simulink model "HDVSim_XNG_Hybrid_Truck"  (R2019b)
settings: Solver=ode3  StartTime=0.0  StopTime=inf  FixedStep=sample_time  MaxStep=0.1  RelTol=1e-3  EnableMultiTasking=on
model workspace (MAT data in the model file):
  Anschaffungskosten = [3707764736; 2; 1; 1; 1; 1]
  Bat.Useable_capacity = 27
  Bat.Useable_range = 0.32
  Bat.Type = 2
  Bat.SOC_start = 1
  Bat.Voltage = 800
  Bat.Charge_cycles = 13055.4976829
  Bat.Efficiency_power_electronics = 0.95
  DrvTrn = 4
  Eigenschaften = [3707764736; 2; 1; 1; 2; 2]
  Electric_Truck = 0
  Elektro_LKW = 0
  Fahrzyklus = 5
  Fueltype = 6
  Gewichte = [3707764736; 2; 1; 1; 3; 3]
  Hybrid_LKW = 1
  Hybrid_Truck = 1
  IZ12 = 3600
  Kosten = [3707764736; 2; 1; 1; 3; 3]
  Kraftstoff = 6
  SOC_T_EM_completely_available = 0.448
  SOC_addition = 0.2
  SOC_soll = 0.5
  SOC_target = 0.05
  Sailing_without_drag_torque = 0
  TCO = TCO
  TCO.Ratio_Trailer_Truck = [1 1]
  TCO.Purchase_price = [113872 59191]
  TCO.Price_increase_repurchase = [3.5 3.5]
  TCO.Replacement_original_price = [77010 54183]
  TCO.Residual_value = [34162 0]
  TCO.bDiesel = 0
  TCO.CNG_consumption = 0
  TCO.LNG_consumption = 23.6968877169
  TCO.energyTotal = 19.9299848679
  TCO.Hydrogen_consumption = 0
  TCO.Tire_costs_set = [2700 5008]
  TCO.Amortization_value = [94665 81874]
  TCO.Toll_rate = 0.135
  TCO.Daily_working_time = 8
  TCO.Other_works_day = 0.5
  TCO.Gross_hourly_wage_driver = 13
  TCO.Operating_days_year = 240
  TCO.Annual_gross_wage_driver = 24960
  TCO.Personnel_factor = 1.3
  TCO.Annual_gross_wage_truck = 32448
  TCO.Social_costs_percent = 25.75
  TCO.Social_costs_EUR = 8355
  TCO.Back_up_driver = 0
  TCO.Fees_day = 12
  TCO.Fees_year = 2880
  TCO.Driver_costs_other = 0
  TCO.Driving_personnel_costs_total = 43683
  TCO.Diesel_price = 0.95
  TCO.Diesel_price_external = 1
  TCO.Adblue_price = 0.68
  TCO.CNG_price = 0.88
  TCO.LNG_price = 0.81
  TCO.Electricity_price = 0.12
  TCO.Hydrogen_price = 9
  TCO.Interest_current_asset = 8.4
  TCO.Interest_fixed_asset = 8.4
  TCO.Administrative_costs = 10.7
  TCO.Driving_distance = 108.251724403
  TCO.Ratio_toll_route = 75
  TCO.Loading_time_combination = 0.25
  TCO.Unloading_time_combination = 0.25
  TCO.Ratio_empty_trips = 0
  TCO.Average_speed = 78.4432785528
  TCO.Daily_driving_time = 5.51
  TCO.Annual_mileage = [103733 103733]
  TCO.Operating_life = [6 12]
  TCO.Tire_mileage = [140000 170000]
  TCO.Ratio_inhouse_tanking_diesel = 75
  TCO.Lubricant_consumption = 1
  TCO.Adblue_consumption = 4
  TCO.Afa_time_dependent = [100 100]
  TCO.Afa_power_dependent = [0 0]
  TCO.Current_asset = [6000 1400]
  TCO.Taxes = [556 373]
  TCO.Insurance_vehicle = [6298 677]
  TCO.Insurance_transport = 1000
  TCO.Communication = 600
  TCO.Repair_maintenance_care = [0.13 0.025]
  TCO.Amortisation_time_dependent = [15778 6823]
  TCO.Current_assets_interest_bearing = 622
  TCO.Fixed_assets_interest_bearing = [4783 2486]
  TCO.Insurance = [7298 677]
  TCO.Sum_vehicle_costs = [29637 10359]
  TCO.Sum_fix_costs = [73320 10359]
  TCO.Amortisation_performance_related = [0 0]
  TCO.Fuel_costs = 22392
  TCO.Lubricant_costs = 199
  TCO.Adblue_costs = 0
  TCO.Repair_costs = [13485 2593]
  TCO.Tire_costs = [2001 3056]
  TCO.Lebensdauer_Batteriepack = 7.12416466393
  TCO.Anschaffungspreis_Akkupack = 19520.8885872
  TCO.Verschleisskosten_Batteriepack = 0
  TCO.Sum_variable_costs = [38077 5649]
  TCO.Sum_fix_variable_costs = [111397 16008]
  TCO.Overhead_costs = 15266
  TCO.Toll_costs = 10502.96625
  TCO.Total_costs = 153173.96625
  TCO.Results = table
  TCO.Results.data = {{[]; []; []; []; []; []; []; []; []; []; []; []; []; []; []; []; []; []; []; []; []; []; []}, {[]; 0.217876664128; 0.00599616322675; 0.070074132629; 0.0089556843049; 0.0768800670953; 0.00578408028303; 0.385566791667; 0.421109965006; 0.806676756673; []; 0; 0.215861876163; 0; 0.154994071318; 0.00191838662721; 0.0487501566522; 0.42152449076; []; 1.22820124743; 0.147166282668; 0.10125; 1.4766175301}, {[]; []; []; []; []; []; []; []; []; []; []; []; []; []; []; []; []; []; []; []; []; []; []}, {'#cccccc'; '#9DFF00'; '#CEFF00'; '#FFFF00'; '#FFDF00'; '#FFB900'; '#FF9200'; '#FF5D00'; '#FF7F7F'; '#FF2900'; '#cccccc'; '#00FF85'; '#00FFC6'; '#00FFF2'; '#00FFFF'; '#00DBFF'; '#00B3FF'; '#0088FF'; '#cccccc'; '#B100FF'; '#FF00FF'; '#FF00C0'; '#FF0070'}, [0; 1; 1; 1; 1; 1; 1; 0; 1; 0; 0; 1; 1; 1; 1; 1; 1; 0; 0; 0; 1; 1; 0], {[]; 22601; 622; 7269; 929; 7975; 600; 39996; 43683; 83679; []; 0; 22392; 0; 16078; 199; 5057; 43726; []; 127405; 15266; 10502.96625; 153173.96625}}
  TCO.Results.ndims = 2
  TCO.Results.nrows = 23
  TCO.Results.rownames = {'Time_dependent_vehicle_costs'; 'Amortisation_time_dependent'; 'Current_assets_interest_bearing'; 'Fixed_assets_interest_bearing'; 'Taxes'; 'Insurance'; 'Communication'; 'Sum_vehicle_costs'; 'Driving_personnel_costs_total'; 'Sum_fix_costs'; 'Performance_related_vehicle_costs'; 'Amortisation_performance_related'; 'Fuel_costs'; 'Adblue_costs'; 'Repair_costs'; 'Lubricant_costs'; 'Tire_costs'; 'Sum_variable_costs'; 'Sum_costs'; 'Sum_fix_variable_costs'; 'Overhead_costs'; 'Toll_costs'; 'Total_costs'}
  TCO.Results.nvars = 6
  TCO.Results.varnames = {'Euro_Year', 'Euro_km', 'Percent', 'Cellcolor', 'Diagramm', 'Euro_Jahr'}
  TCO.Results.props.CustomProps = struct()
  TCO.Results.props.VariableCustomProps = struct()
  TCO.Results.props.versionSavedFrom = 4
  TCO.Results.props.minCompatibleVersion = 1
  TCO.Results.props.incompatibilityMsg = ''
  TCO.Results.props.VersionSavedFrom = 4
  TCO.Results.props.Description = ''
  TCO.Results.props.useVariableNamesOriginal = 0
  TCO.Results.props.useDimensionNamesOriginal = 0
  TCO.Results.props.VariableNamesOriginal = {}
  TCO.Results.props.DimensionNames = {'Row', 'Variables'}
  TCO.Results.props.DimensionNamesOriginal = {}
  TCO.Results.props.UserData = []
  TCO.Results.props.VariableDescriptions = {}
  TCO.Results.props.VariableUnits = {}
  TCO.Results.props.VariableContinuity = {}
  TCO_Anhaenger = 1
  TCO_Trailer = 1
  T_distance_LPS_down = 141
  T_distance_LPS_up = 366
  VSim.Display = 3
  VSim.Opt = 0
  VSim.v_t = [0 0 0 0 0 0 7.78702558964 13.5455486475 17.5098763901 20.64771434 22.6611629891 24.5808920247 26.497930615 27.9436285122 29.2700076923 30.5941752972 31.916035258 33.1908328261 34.0616736486 34.9308240914 35.7982444287 36.663895271 37.5277375709 38.3897326301 39.2498421055 40.1080280155 40.9642527457 41.8184790552 42.6706700821 43.4527673116 43.9611797576 44.4683183246 44.9741715644 45.4787281689 45.9819769713 46.4839069461 47.9488358042 49.5523927745 51.1896098137 52.8609582212 54.5660311627 56.2956841938 58.0503332316 59.8108324205 61.5662383358 63.3222898223 65.0739909595 66.8139377448 68.5280042906 70.2103693282 71.8624252942 73.4760089439 75.0504359591 76.5862765506 78.0850203676 79.5458647769 80.9710388101 81.5966525882 80.8908314832 81.9503579711 83.0914322002 84.2274160693 85.3582506742 86.2443475854 86.4930083388 86.555730356 86.5740585846]
  Vehicle = 'LNGHybrid'
  WPT.Voltage = 0
  WPT.P_max = 200
  WPT.expansion = 0.3
  WPT.eta = 0.92
  WPT.SOC_target = 0.8
  WPT.SOC_electric_only = 0.3
  Zugmaschinengewicht = 0
  acquisitionCosts = acquisitionCosts
  acquisitionCosts.KA_ZM = 113872.311812
  acquisitionCosts.KG = 5933.68525734
  acquisitionCosts.Getr_m = 256.411373638
  acquisitionCosts.KMot = 9881.5
  acquisitionCosts.Motor_Mmax = 1500
  acquisitionCosts.Motor_m = 846.7
  acquisitionCosts.KH = 15531.6957356
  acquisitionCosts.Hybrid = 1
  acquisitionCosts.Bat = 12080
  acquisitionCosts.Bat_type = 2
  acquisitionCosts.Bat_m = 419.254658385
  acquisitionCosts.Bat_kWh = 67.5
  acquisitionCosts.Ratio_BEV2PHEV = 1
  acquisitionCosts.EM = 1927.38569435
  acquisitionCosts.EM_Type = 2
  acquisitionCosts.EM_P_max = 187.306675836
  acquisitionCosts.LE = 842.88004126
  acquisitionCosts.KWPT = 10000
  acquisitionCosts.FC = 0
  acquisitionCosts.P_FC = 0
  acquisitionCosts.KA = 1563
  acquisitionCosts.v_diesel = 0
  acquisitionCosts.v_cng = 0
  acquisitionCosts.v_lng = 400
  acquisitionCosts.m_h2 = 0
  acquisitionCosts.KT = 4692.4
  acquisitionCosts.KTD = 0
  acquisitionCosts.KTCNG = 0
  acquisitionCosts.KTLNG = 4692.4
  acquisitionCosts.KTFC = 0
  acquisition_costs = [3707764736; 2; 1; 1; 3; 3]
  addition_for_critical_slope = 0.037
  ambient.gravity = 9.80665
  ambient.air_density = 1.1883
  ambient.friction_coeff = 0.8
  auxiliary_consumers.onBattery = 1
  auxiliary_consumers.power = 4859
  critical_altitude_difference = 50
  cycle.distance = [0 2e-05 0.25 0.6036 1.1529 1.9121 2.8899 4.092 5.1928 6.1952 7.4225 8.8723 ... (10385 elements, 10385x1)]
  cycle.speed = [0 0 16.6666666667 16.6666666667 16.6666666667 16.6666666667 16.6666666667 16.6666666667 16.6666666667 16.6666666667 16.6666666667 16.6666666667 ... (10385 elements, 10385x1)]
  cycle.slope = [0.029502 0.029502 0.029502 0.029502 0.029502 0.029502 0.029502 0.029502 0.029502 0.029502 0.029502 0.029502 ... (10385 elements, 10385x1)]
  cycle.stop_time = [1 1 0 0 0 0 0 0 0 0 0 0 ... (10385 elements, 10385x1)]
  cycle.altitude = [0 0 0 0 0 0 0 0 0 0 0 0 ... (10385 elements, 10385x1)]
  cycle.stop_end = 5
  cycle.stop_start = 5
  cycle.speed_init = 0
  cycle.delta12 = [0 0 0 0 0 0 0 0 0 0 0 0 ... (10385 elements, 1x10385)]
  cycle.delta23 = [0 0 0 0 0 0 0 0 0 0 0 0 ... (10385 elements, 1x10385)]
  dcycle = 20
  distance_1_PPC = 330
  distance_2_PPC = 340
  distance_PPC_altitude_difference = 4601
  driver.controller_proportional_gain = 230000
  driver.controller_integral_gain = 3000
  driver.slew_rate_throttle_in = 2
  driver.slew_rate_throttle_out = -2
  driver.slew_rate_brake_in = 2
  driver.slew_rate_brake_out = -2
  em.P_max = 187.306675836
  em.M_max = 1211
  em.n_eck = 1477
  em.n_max = 2000
  em.shift_parameter.n_pref = 1388.38
  em.shift_parameter.n2 = 310.17
  em.shift_parameter.n6 = 1600
  em.shift_parameter.M_max = 1211
  em.fuel.co2_per_kwh = 504
  em.speed = [0 10 20 30 40 50 60 70 80 90 100 110 120 130 140 150 160 170 180 190 200 210 220 230 240 250 260 270 280 290 300 310 320 330 340 350 360 370 380 390 400 410 420 430 440 450 460 470 480 490 500 510 520 530 540 550 560 570 580 590 600 610 620 630 640 650 660 670 680 690 700 710 720 730 740 750 760 770 780 790 800 810 820 830 840 850 860 870 880 890 900 910 920 930 940 950 960 970 980 990 1000 1010 1020 1030 1040 1050 1060 1070 1080 1090 1100 1110 1120 1130 1140 1150 1160 1170 1180 1190 1200 1210 1220 1230 1240 1250 1260 1270 1280 1290 1300 1310 1320 1330 1340 1350 1360 1370 1380 1390 1400 1410 1420 1430 1440 1450 1460 1470 1480 1490 1500 1510 1520 1530 1540 1550 1560 1570 1580 1590 1600 1610 1620 1630 1640 1650 1660 1670 1680 1690 1700 1710 1720 1730 1740 1750 1760 1770 1780 1790 1800 1810 1820 1830 1840 1850 1860 1870 1880 1890 1900 1910 1920 1930 1940 1950 1960 1970 1980 1990 2000]
  em.trq = [1211 1211 1211 1211 1211 1211 1211 1211 1211 1211 1211 1211 1211 1211 1211 1211 1211 1211 1211 1211 1211 1211 1211 1211 1211 1211 1211 1211 1211 1211 1211 1211 1211 1211 1211 1211 1211 1211 1211 1211 1211 1211 1211 1211 1211 1211 1211 1211 1211 1211 1211 1211 1211 1211 1211 1211 1211 1211 1211 1211 1211 1211 1211 1211 1211 1211 1211 1211 1211 1211 1211 1211 1211 1211 1211 1211 1211 1211 1211 1211 1211 1211 1211 1211 1211 1211 1211 1211 1211 1211 1211 1211 1211 1211 1211 1211 1211 1211 1211 1211 1211 1211 1211 1211 1211 1211 1211 1211 1211 1211 1211 1211 1211 1211 1211 1211 1211 1211 1211 1211 1211 1211 1211 1211 1211 1211 1211 1211 1211 1211 1211 1211 1211 1211 1211 1211 1211 1211 1211 1211 1211 1211 1211 1211 1211 1211 1211 1211 1208.54527027 1200.43422819 1192.43133333 1184.53443709 1176.74144737 1169.0503268 1161.45909091 1153.96580645 1146.56858974 1139.2656051 1132.05506329 1124.93522013 1117.904375 1110.96086957 1104.10308642 1097.32944785 1090.63841463 1084.02848485 1077.49819277 1071.04610778 1064.67083333 1058.37100592 1052.14529412 1045.99239766 1039.91104651 1033.9 1027.95804598 1022.084 1016.27670455 1010.53502825 1004.85786517 999.244134078 993.692777778 988.202762431 982.773076923 977.40273224 972.09076087 966.836216216 961.638172043 956.495721925 951.407978723 946.374074074 941.393157895 936.464397906 931.586979167 926.760103627 921.982989691 917.254871795 912.575 907.942639594 903.357070707 898.81758794 894.3235]
  em.Type = 2
  em.efficiency.characteristic_map = [0.001 0.001 0.001 0.001 0.001 0.001 0.001 0.001 0.001 0.001 0.001 0.001 ... (20301 elements, 101x201)]
  em.efficiency.speed = [0 10 20 30 40 50 60 70 80 90 100 110 120 130 140 150 160 170 180 190 200 210 220 230 240 250 260 270 280 290 300 310 320 330 340 350 360 370 380 390 400 410 420 430 440 450 460 470 480 490 500 510 520 530 540 550 560 570 580 590 600 610 620 630 640 650 660 670 680 690 700 710 720 730 740 750 760 770 780 790 800 810 820 830 840 850 860 870 880 890 900 910 920 930 940 950 960 970 980 990 1000 1010 1020 1030 1040 1050 1060 1070 1080 1090 1100 1110 1120 1130 1140 1150 1160 1170 1180 1190 1200 1210 1220 1230 1240 1250 1260 1270 1280 1290 1300 1310 1320 1330 1340 1350 1360 1370 1380 1390 1400 1410 1420 1430 1440 1450 1460 1470 1480 1490 1500 1510 1520 1530 1540 1550 1560 1570 1580 1590 1600 1610 1620 1630 1640 1650 1660 1670 1680 1690 1700 1710 1720 1730 1740 1750 1760 1770 1780 1790 1800 1810 1820 1830 1840 1850 1860 1870 1880 1890 1900 1910 1920 1930 1940 1950 1960 1970 1980 1990 2000]
  em.efficiency.torque = [0 12.11 24.22 36.33 48.44 60.55 72.66 84.77 96.88 108.99 121.1 133.21 145.32 157.43 169.54 181.65 193.76 205.87 217.98 230.09 242.2 254.31 266.42 278.53 290.64 302.75 314.86 326.97 339.08 351.19 363.3 375.41 387.52 399.63 411.74 423.85 435.96 448.07 460.18 472.29 484.4 496.51 508.62 520.73 532.84 544.95 557.06 569.17 581.28 593.39 605.5 617.61 629.72 641.83 653.94 666.05 678.16 690.27 702.38 714.49 726.6 738.71 750.82 762.93 775.04 787.15 799.26 811.37 823.48 835.59 847.7 859.81 871.92 884.03 896.14 908.25 920.36 932.47 944.58 956.69 968.8 980.91 993.02 1005.13 1017.24 1029.35 1041.46 1053.57 1065.68 1077.79 1089.9 1102.01 1114.12 1126.23 1138.34 1150.45 1162.56 1174.67 1186.78 1198.89 1211]
  em.noEM = 2
  em_M_max = 797.415556
  engine.bsfc.be = [0.131482188876 0.133620299042 0.135839892639 0.138147190922 0.140549191494 0.143053789211 0.145669914251 0.148407687189 0.151278588529 0.154295635515 0.157473550327 0.160828886947 ... (38000 elements, 190x200)]
  engine.bsfc.trqorig = [0 5.95509049885 11.9101809977 17.8652714966 23.8203619954 29.7754524943 35.7305429931 41.685633492 47.6407239908 53.5958144897 59.5509049885 65.5059954874 ... (190 elements, 190x1)]
  engine.bsfc.speed = [798.227848101 805.263023981 812.29819986 819.333375739 826.368551619 833.403727498 840.438903378 847.474079257 854.509255136 861.544431016 868.579606895 875.614782775 ... (200 elements, 1x200)]
  engine.bsfc.be_min = 0.372504791257
  engine.bsfc.M_be_min_orig = [684.835407368 690.790497867 696.745588366 702.700678865 708.655769363 714.610859862 720.565950361 732.476131359 738.431221858 744.386312357 750.341402855 756.296493354 ... (200 elements, 1x200)]
  engine.bsfc.trq = [0 7.97556763239 15.9511352648 23.9267028972 31.9022705296 39.877838162 47.8534057943 55.8289734267 63.8045410591 71.7801086915 79.7556763239 87.7312439563 ... (190 elements, 190x1)]
  engine.bsfc.M_be_min = [917.190277725 925.165845357 933.14141299 941.116980622 949.092548255 957.068115887 965.043683519 980.994818784 988.970386417 996.945954049 1004.92152168 1012.89708931 ... (200 elements, 1x200)]
  engine.full_load.trqorig = [684.835407368 690.790497867 696.745588366 702.700678865 708.655769363 714.610859862 720.565950361 732.476131359 738.431221858 744.386312357 750.341402855 756.296493354 ... (200 elements, 1x200)]
  engine.full_load.speed = [798.227848101 805.263023981 812.29819986 819.333375739 826.368551619 833.403727498 840.438903378 847.474079257 854.509255136 861.544431016 868.579606895 875.614782775 ... (200 elements, 1x200)]
  engine.full_load.trq = [917.190277725 925.165845357 933.14141299 941.116980622 949.092548255 957.068115887 965.043683519 980.994818784 988.970386417 996.945954049 1004.92152168 1012.89708931 ... (200 elements, 1x200)]
  engine.full_load.power = [76.6681414837 78.0164109099 79.3764318868 80.7482044142 82.1317284922 83.5270041209 84.9340313002 87.0606199483 88.4970260041 89.9451836104 91.4050927674 92.876753475 ... (200 elements, 1x200)]
  engine.number = 3
  engine.M_max_original = 1120
  engine.M_max = 1500
  engine.scale_factor = 1.33928571429
  engine.speed_min = 800
  engine.speed_max = 1900
  engine.idle_fuel_consumption = 1.5
  engine.drag_torque.trq = [-160.714285714 -348.214285714]
  engine.drag_torque.speed = [600 2190]
  engine.shift_parameter.n_lo = 1000
  engine.shift_parameter.n_pref = 1279
  engine.shift_parameter.n1 = 600
  engine.shift_parameter.n2 = 800
  engine.shift_parameter.n3 = 1139.5
  engine.shift_parameter.n4 = 800
  engine.shift_parameter.n5 = 1279
  engine.shift_parameter.n6 = 1900
  engine.shift_parameter.M_max = 1499.40671489
  engine.fuel.density = 424
  engine.fuel.co2_per_kg = 2.75
  engine.fuel.heat_of_combustion = 50000
  engine.fuel.Gasart = 'LNG'
  final_drive.ratio = 2.884
  final_drive.trq_eff = 0.98
  ifElectric = 0
  ifOptimized = 1
  look_ahead_PPC = 710
  max_distance = 108191.00003
  name = 'HDVSim_XNG_Hybrid_Truck'
  preditiveCruiseControl = 0
  propertie = [3707764736; 2; 1; 1; 4; 4]
  prospective_cruise_control = 1
  sample_time = 0.1
  tank.v_diesel = 0
  tank.v_cng = 0
  tank.v_lng = 400
  tank.m_h2 = 0
  tires.radius = 0.4465
  tires.roll_drag_coeff.total = 0.007
  transmission.init_gear = 1
  transmission.shift_time = 1
  transmission.ratios = [11.689 8.8947844856 6.76851664345 5.15052586455 3.91931025346 2.98241252774 2.26947700243 1.72696627869 1.31414088997 1]
  transmission.trq_eff = [0.96 0.96 0.96 0.96 0.98 0.97 0.97 0.97 0.97 0.99]
  transmission.lamda = [1.10949792808 1.06119976752 1.03288013965 1.02166016924 1.01558545301 1.01166143122 1.00954352608 1.0082007006 1.00714498093 1.006050163]
  transmission.z = 10
  v_PPC_delta = 2.77777777778
  v_max_electrical_drive_only = 12.8888888889
  vehicle.long_truck = 0
  vehicle.payload = 19300
  vehicle.frontal_area = 10.2
  vehicle.air_drag_coeff = 0.55
  vehicle.Fx_max = 90221.18
  vehicle.F_brake_max = 309890.14
  vehicle.mass = 13055.4017043
  vehicleProperties = vehicleProperties
  vehicleProperties.CO2_EM_Range = 100
  vehicleProperties.a_0_80_Range = 54
  vehicleProperties.BOK_ak = 2.55
  vehicleProperties.BOK_Range = 4.65
  vehicleProperties.V_Rel_ak = 0.55
  vehicleProperties.V_Rel_Range = 0.35
  vehicleProperties.VWF_ak = 0.9
  vehicleProperties.VWF_Range = 7.2
  vehicle_F_brake_max = 309890.14
  weights = weights
  weights.m_Engine = 846.7
  weights.m_Gearbox = 256.411373638
  weights.m_Retarder = 82
  weights.m_tank_system = 252.803932883
  weights.m_Fuel = 216
  weights.m_Exhaust = 118.57
  weights.m_Battery = 419.254658385
  weights.m_EM = 208.118528706
  weights.m_PwrElectr = 17.3432107255
  weights.m_Charger = 15
  weights.m_Trailer = 5400
  weights.m_Total = 7655.40170434
  weights.m_Base = 5223.2
  weights.Hybrid = 1
  weights.max_Payload = 27944.5982957
  weights.m_Max = 41000
  x = [11.689 7 0 0 2.884 1500 1000 1279 10 62 24 25 1211 1477 27 0.05 0.448 46.4 366 141 0.037 4601 50 0.2 0.32 2 2]
values from neighbouring files (symbols this model uses):
  transmission = Transmission_gearing(15.86, 7, 0, 0, final_drive.ratio)    [Parameterizing.m]
undefined symbols (not defined in the file or in its folder): Ion, Lithium, Vorausschauender_Tempomat
libraries: simulink x22, powerlib x2, electricdrivelib x1
(part 1 of 3)
settings Model: Creator=Alexander Suessmann  LastModifiedBy=ga73rep
settings DataIOCC: SaveFormat=Array  SignalLoggingSaveFormat=ModelDataLogs  SaveOutput=off  SignalLogging=off  DSMLogging=off  ReturnWorkspaceOutputs=off  SignalLoggingName=datalog
settings OptimizationCC: ExpressionDepthLimit=2147483647  InitFltsAndDblsToZero=on  LifeSpan=inf
settings DebuggingCC: UnderspecifiedInitializationDetection=Classic  MergeDetectMultiDrivingBlocksExec=none  SolverPrmCheckMsg=warning  MultiTaskDSMMsg=warning  MultiTaskCondExecSysMsg=none  FcnCallInpInsideContextMsg=warning  AllowSymbolicDim=off  StateNameClashWarn=warning  SFUnexpectedBacktrackingDiag=warning  SFNoUnconditionalDefaultTransitionDiag=warning  SFExecutionAtInitializationDiag=none  ArithmeticOperatorsInVariantConditions=warning
settings HardwareCC: ProdBitPerPointer=32  ProdBitPerSizeT=32  ProdBitPerPtrDiffT=32  ProdLargestAtomicFloat=None  ProdIntDivRoundTo=Undefined  ProdEndianess=Unspecified  ProdWordSize=32  ProdHWDeviceType=32-bit Generic
settings ModelReferenceCC: PropagateSignalLabelsOutOfModel=off
settings SFSimCC: SimUseLocalCustomCode=off  CustomCodeUndefinedFunction=UseInterfaceOnly
settings RTWCC: RTWUseLocalCustomCode=off
settings CodeAppCC: StateflowObjectComments=on
settings GRTTargetCC: TargetLangStandard=C89/C90 (ANSI)  GeneratePreprocessorConditionals=Disable all  CombineOutputUpdateFcns=off  GRTInterface=on
settings ConfigComp: CovMetricSettings=dw  CovHtmlReporting=on  CovSaveCumulativeToWorkspaceVar=on  CovSaveSingleToWorkspaceVar=on  CovExternalEMLEnable=off
settings SimscapeCC: SimscapeNormalizeSystem=off

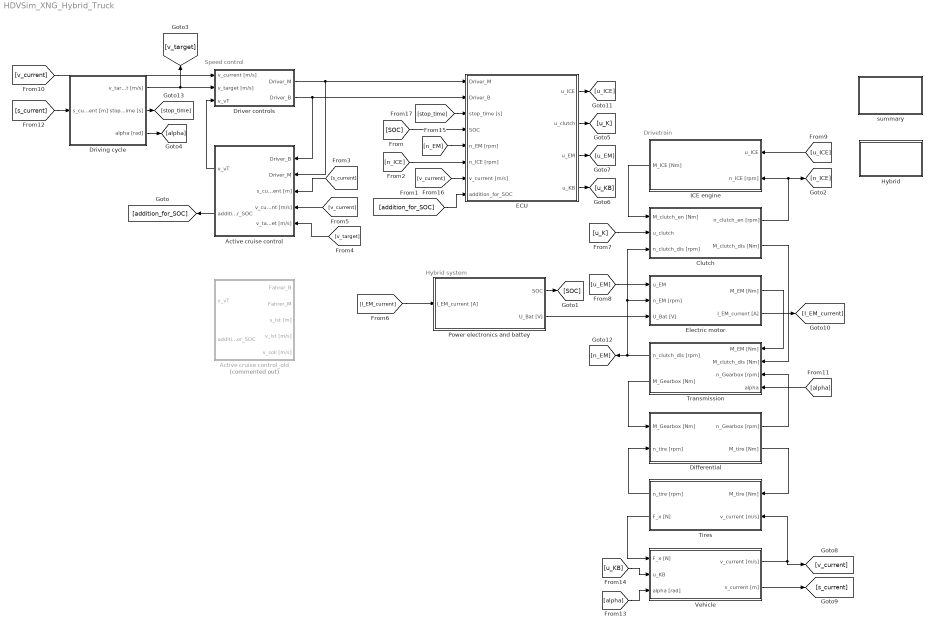
== system HDVSim_XNG_Hybrid_Truck ==
  note: Speed control
  note: Drivetrain
  note: Hybrid system
  Active cruise control : Subsystem
  Active cruise control_old : Subsystem  Commented=on
  Clutch : Subsystem
  Differential : Subsystem
  Driver controls : Subsystem
  Driving cycle : Subsystem
  ECU : Subsystem
  Electric motor : Subsystem
  Hybrid : Subsystem
  ICE engine : Subsystem
  Power electronics and battey : Subsystem
  Tires : Subsystem
  Transmission : Subsystem
  Vehicle : Subsystem
  summary : Subsystem
  wires:
  ICE engine/1(M_ICE [Nm]) -> Clutch/1(M_clutch_en [Nm])
  Transmission/1(n_clutch_dis [rpm]) -> [n_EM], Clutch/3(n_clutch_dis [rpm]), Electric motor/2(n_EM [rpm])
  Transmission/2(M_Gearbox [Nm]) -> Differential/1(M_Gearbox [Nm])
  Tires/1(n_tire [rpm]) -> Differential/2(n_tire [rpm])
  Clutch/1(n_clutch_en [rpm]) -> [n_ICE], ICE engine/2(n_ICE [rpm])
  Clutch/2(M_clutch_dis [Nm]) -> Transmission/2(M_clutch_dis [Nm])
  Differential/1(n_Gearbox [rpm]) -> Transmission/3(n_Gearbox [rpm])
  Differential/2(M_tire [Nm]) -> Tires/1(M_tire [Nm])
  Vehicle/1(v_current [m/s]) -> [v_current], Tires/2(v_current [m/s])
  Tires/2(F_x [N]) -> Vehicle/1(F_x [N])
  Electric motor/1(M_EM [Nm]) -> Transmission/1(M_EM [Nm])
  Driving cycle/1(v_target [m/s]) -> [v_target], Driver controls/2(v_target [m/s])
  Driving cycle/3(alpha [rad]) -> [alpha]
  Active cruise control/2(addition_for_SOC) -> [addition_for_SOC]
  Active cruise control/1(v_vT) -> Driver controls/3(v_vT)
  [s_current] -> Driving cycle/1(s_current [m])
  [v_current] -> Driver controls/1(v_current [m/s])
  [n_ICE] -> ECU/6(n_ICE [rpm])
  [SOC] -> ECU/4(SOC)
  [n_EM] -> ECU/5(n_EM [rpm])
  [v_current] -> ECU/7(v_current [m/s])
  [addition_for_SOC] -> ECU/8(addition_for_SOC)
  Driver controls/1(Driver_M) -> Active cruise control/2(Driver_M), ECU/1(Driver_M)
  Driver controls/2(Driver_B) -> Active cruise control/1(Driver_B), ECU/2(Driver_B)
  [s_current] -> Active cruise control/3(s_current [m])
  [v_current] -> Active cruise control/4(v_current [m/s])
  [v_target] -> Active cruise control/5(v_target [m/s])
  [I_EM_current] -> Power electronics and battey/1(I_EM_current [A])
  ECU/1(u_ICE) -> [u_ICE]
  ECU/2(u_clutch) -> [u_K]
  ECU/3(u_EM) -> [u_EM]
  ECU/4(u_KB) -> [u_KB]
  [u_K] -> Clutch/2(u_clutch)
  [u_EM] -> Electric motor/1(u_EM)
  Power electronics and battey/1(SOC) -> [SOC]
  Power electronics and battey/2(U_Bat [V]) -> Electric motor/3(U_Bat [V])
  [u_ICE] -> ICE engine/1(u_ICE)
  Electric motor/2(I_EM_current [A]) -> [I_EM_current]
  [alpha] -> Transmission/4(alpha)
  Vehicle/2(s_current [m]) -> [s_current]
  [u_KB] -> Vehicle/2(u_KB)
  [alpha] -> Vehicle/3(alpha [rad])
  Driving cycle/2(stop_time [s]) -> [stop_time]
  [stop_time] -> ECU/3(stop_time [s])

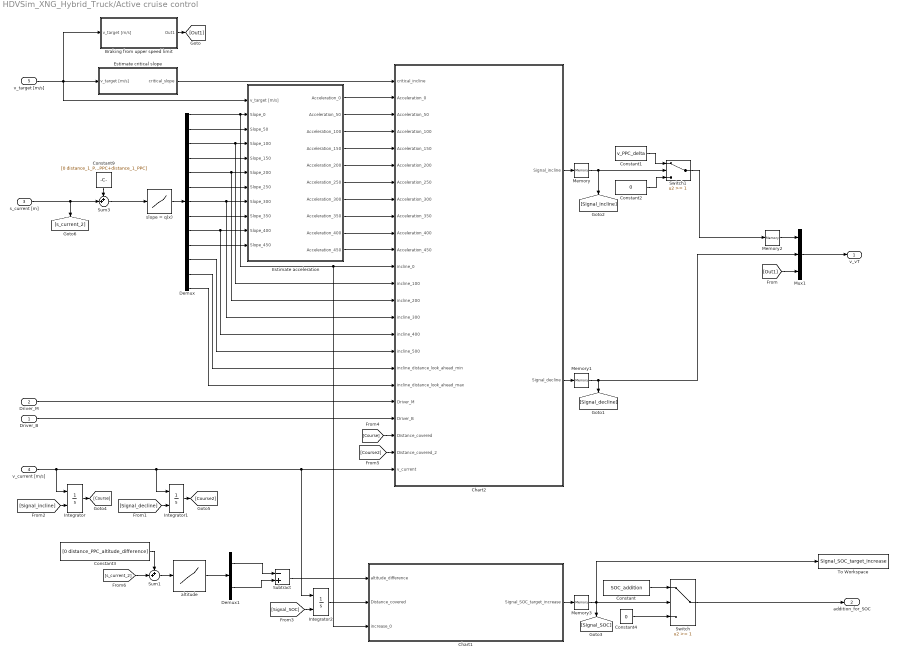
== system HDVSim_XNG_Hybrid_Truck/Active cruise control ==
  Driver_B : Inport
  Driver_M : Inport  Port=2
  s_current [m] : Inport  Port=3
  v_current [m/s] : Inport  Port=4
  v_target [m/s] : Inport  Port=5
  Braking from upper speed limit : Subsystem
  Chart1 : Stateflow chart  TreatAsAtomicUnit=on
  Chart2 : Stateflow chart  TreatAsAtomicUnit=on
  Constant : Constant  Value=SOC_addition
  Constant1 : Constant  Value=v_PPC_delta
  Constant2 : Constant  Value=0
  Constant3 : Constant  Value=[0 distance_PPC_altitude_difference]
  Constant4 : Constant  Value=0
  Constant9 : Constant  Value=[0 distance_1_PPC/5 2*distance_1_PPC/5 3*distance_1_PPC/5 4*distance_1_PPC/5 distance_1_PPC 6*distance_1_PPC/5 7*distance_1_PPC/5 8*distance_1_PPC/5 9*distance_1_PPC/5 10*distance_1_PPC/5 look_ahead_PPC look_ahead_PPC+distance_1_PPC]
  Demux : Demux  Outputs=13
  Demux1 : Demux  Outputs=2
  Estimate acceleration : Subsystem
  Estimate critical slope : Subsystem
  Integrator : Integrator  ExternalReset=rising
  Integrator1 : Integrator  ExternalReset=rising
  Integrator2 : Integrator  ExternalReset=rising
  Memory{,1..3} (4 alike) : Memory
  Mux1 : Mux  Inputs=3
  Subtract : Sum  Inputs=-+
  Sum1 : Sum  Inputs=++|
  Sum3 : Sum  Inputs=++|
  Switch : Switch  Threshold=1
  Switch1 : Switch  Threshold=1
  To Workspace : ToWorkspace  VariableName=Signal_SOC_target_increase  SaveFormat=Structure With Time  SampleTime=-1
  altitude : Lookup_n-D  NumberOfTableDimensions=1  BreakpointsForDimension1=cycle.distance  Table=cycle.altitude  InterpMethod=Flat  RndMeth=Simplest  InputSameDT=on
  slope = q(x) : Lookup  InputValues=cycle.distance  Table=cycle.slope  LookUpMeth=Interpolation-Use End Values
  v_vT : Outport  VectorParamsAs1DForOutWhenUnconnected=off
  addition_for_SOC : Outport  Port=2  VectorParamsAs1DForOutWhenUnconnected=off
  wires:
  slope = q(x)/1 -> Demux/1
  Demux/1 -> Chart1/3(increase_0), Chart2/12(incline_0), Estimate acceleration/2(Slope_0)
  Estimate critical slope/1(critical_slope) -> Chart2/1(critical_incline)
  Chart2/1(Signal_incline) -> Memory/1
  v_current [m/s]/1 -> Integrator/1, Integrator1/1, Chart2/24(v_current), Integrator2/1
  Memory/1 -> [Signal_incline], Switch1/2
  Mux1/1 -> v_vT/1
  Constant9/1 -> Sum3/1
  Sum3/1 -> slope = q(x)/1
  Estimate acceleration/1(Acceleration_0) -> Chart2/2(Acceleration_0)
  Estimate acceleration/2(Acceleration_50) -> Chart2/3(Acceleration_50)
  Estimate acceleration/3(Acceleration_100) -> Chart2/4(Acceleration_100)
  Estimate acceleration/4(Acceleration_150) -> Chart2/5(Acceleration_150)
  Estimate acceleration/5(Acceleration_200) -> Chart2/6(Acceleration_200)
  Estimate acceleration/6(Acceleration_250) -> Chart2/7(Acceleration_250)
  Estimate acceleration/7(Acceleration_300) -> Chart2/8(Acceleration_300)
  Estimate acceleration/8(Acceleration_350) -> Chart2/9(Acceleration_350)
  Chart2/2(Signal_decline) -> Memory1/1
  Memory1/1 -> Mux1/2, [Signal_decline]
  Driver_B/1 -> Chart2/21(Driver_B)
  Estimate acceleration/9(Acceleration_400) -> Chart2/10(Acceleration_400)
  Estimate acceleration/10(Acceleration_450) -> Chart2/11(Acceleration_450)
  Driver_M/1 -> Chart2/20(Driver_M)
  Demux/2 -> Estimate acceleration/3(Slope_50)
  Demux/3 -> Chart2/13(incline_100), Estimate acceleration/4(Slope_100)
  Demux/4 -> Estimate acceleration/5(Slope_150)
  Demux/5 -> Chart2/14(incline_200), Estimate acceleration/6(Slope_200)
  Demux/6 -> Estimate acceleration/7("Slope_250 ")
  Demux/7 -> Chart2/15(incline_300), Estimate acceleration/8(Slope_300)
  Demux/8 -> Estimate acceleration/9(Slope_350)
  Demux/9 -> Chart2/16(incline_400), Estimate acceleration/10(Slope_400)
  Demux/10 -> Estimate acceleration/11(Slope_450)
  Demux/12 -> Chart2/18(incline_distance_look_ahead_min)
  Demux/13 -> Chart2/19(incline_distance_look_ahead_max)
  v_target [m/s]/1 -> Braking from upper speed limit/1(v_target [m/s]), Estimate acceleration/1(v_target [m/s]), Estimate critical slope/1(v_target [m/s])
  Memory2/1 -> Mux1/1
  Constant1/1 -> Switch1/1
  Constant2/1 -> Switch1/3
  Demux/11 -> Chart2/17(incline_500)
  Switch1/1 -> Memory2/1
  Constant3/1 -> Sum1/1
  s_current [m]/1 -> [s_current_2], Sum3/2
  Sum1/1 -> altitude/1
  altitude/1 -> Demux1/1
  Demux1/2 -> Subtract/2
  Demux1/1 -> Subtract/1
  Subtract/1 -> Chart1/1(altitude_difference)
  Chart1/1(Signal_SOC_target_increase) -> Memory3/1
  Integrator2/1 -> Chart1/2(Distance_covered)
  Constant/1 -> Switch/1
  Constant4/1 -> Switch/3
  Switch/1 -> addition_for_SOC/1
  Memory3/1 -> Switch/2, To Workspace/1, [Signal_SOC]
  [Signal_decline] -> Integrator1/2
  Integrator1/1 -> [Course2]
  Integrator/1 -> [Course]
  [Signal_incline] -> Integrator/2
  [Course] -> Chart2/22(Distance_covered)
  [Course2] -> Chart2/23(Distance_covered_2)
  [Signal_SOC] -> Integrator2/2
  [s_current_2] -> Sum1/2
  Braking from upper speed limit/1(Out1) -> [Out1]
  [Out1] -> Mux1/3

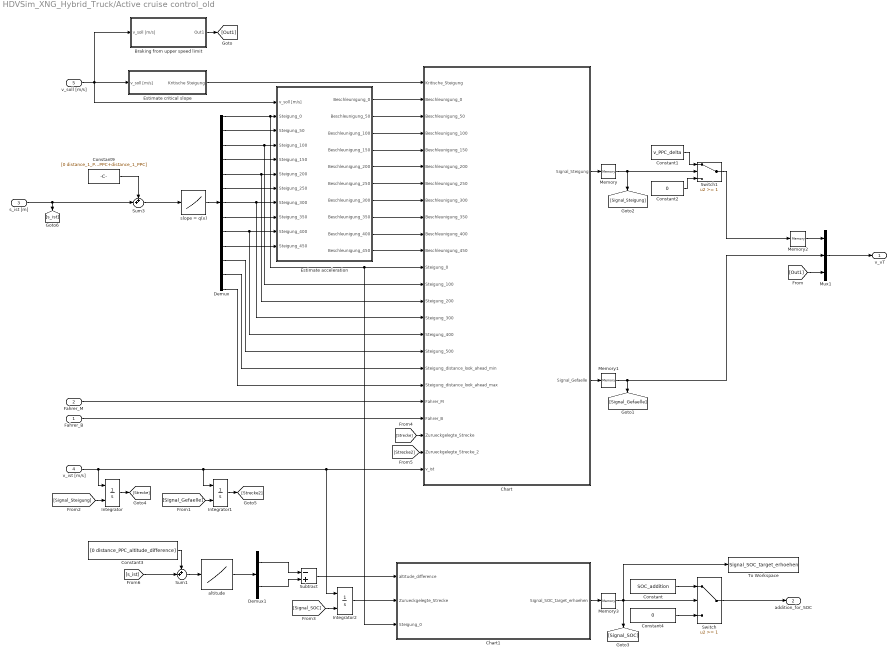
== system HDVSim_XNG_Hybrid_Truck/Active cruise control_old ==
  Fahrer_B : Inport
  Fahrer_M : Inport  Port=2
  s_ist [m] : Inport  Port=3
  v_ist [m/s] : Inport  Port=4
  v_soll [m/s] : Inport  Port=5
  Braking from upper speed limit : Subsystem
  Chart : Stateflow chart  TreatAsAtomicUnit=on
  Chart1 : Stateflow chart  TreatAsAtomicUnit=on
  Constant : Constant  Value=SOC_addition
  Constant1 : Constant  Value=v_PPC_delta
  Constant2 : Constant  Value=0
  Constant3 : Constant  Value=[0 distance_PPC_altitude_difference]
  Constant4 : Constant  Value=0
  Constant9 : Constant  Value=[0 distance_1_PPC/5 2*distance_1_PPC/5 3*distance_1_PPC/5 4*distance_1_PPC/5 distance_1_PPC 6*distance_1_PPC/5 7*distance_1_PPC/5 8*distance_1_PPC/5 9*distance_1_PPC/5 10*distance_1_PPC/5 look_ahead_PPC look_ahead_PPC+distance_1_PPC]
  Demux : Demux  Outputs=13
  Demux1 : Demux  Outputs=2
  Estimate acceleration : Subsystem
  Estimate critical slope : Subsystem
  Integrator : Integrator  ExternalReset=rising
  Integrator1 : Integrator  ExternalReset=rising
  Integrator2 : Integrator  ExternalReset=rising
  Memory{,1..3} (4 alike) : Memory
  Mux1 : Mux  Inputs=3
  Subtract : Sum  Inputs=-+
  Sum1 : Sum  Inputs=++|
  Sum3 : Sum  Inputs=++|
  Switch : Switch  Threshold=1
  Switch1 : Switch  Threshold=1
  To Workspace : ToWorkspace  VariableName=Signal_SOC_target_erhoehen  SaveFormat=Structure With Time  SampleTime=-1
  altitude : Lookup_n-D  NumberOfTableDimensions=1  BreakpointsForDimension1=cycle.distance  Table=cycle.altitude  InterpMethod=Flat  RndMeth=Simplest  InputSameDT=on
  slope = q(x) : Lookup  InputValues=cycle.distance  Table=cycle.slope  LookUpMeth=Interpolation-Use End Values
  v_vT : Outport  VectorParamsAs1DForOutWhenUnconnected=off
  addition_for_SOC : Outport  Port=2  VectorParamsAs1DForOutWhenUnconnected=off
  wires:
  slope = q(x)/1 -> Demux/1
  Demux/1 -> Chart1/3(Steigung_0), Chart/12(Steigung_0), Estimate acceleration/2(Steigung_0)
  Estimate critical slope/1(Kritische Steigung) -> Chart/1(Kritische_Steigung)
  Chart/1(Signal_Steigung) -> Memory/1
  v_ist [m/s]/1 -> Integrator2/1, Chart/24(v_ist), Integrator1/1, Integrator/1
  Memory/1 -> Switch1/2, [Signal_Steigung]
  Mux1/1 -> v_vT/1
  Constant9/1 -> Sum3/1
  Sum3/1 -> slope = q(x)/1
  Estimate acceleration/1(Beschleunigung_0) -> Chart/2(Beschleunigung_0)
  Estimate acceleration/2(Beschleunigung_50) -> Chart/3(Beschleunigung_50)
  Estimate acceleration/3(Beschleunigung_100) -> Chart/4(Beschleunigung_100)
  Estimate acceleration/4(Beschleunigung_150) -> Chart/5(Beschleunigung_150)
  Estimate acceleration/5(Beschleunigung_200) -> Chart/6(Beschleunigung_200)
  Estimate acceleration/6(Beschleunigung_250) -> Chart/7(Beschleunigung_250)
  Estimate acceleration/7(Beschleunigung_300) -> Chart/8(Beschleunigung_300)
  Estimate acceleration/8(Beschleunigung_350) -> Chart/9(Beschleunigung_350)
  Chart/2(Signal_Gefaelle) -> Memory1/1
  Memory1/1 -> Mux1/2, [Signal_Gefaelle]
  Fahrer_B/1 -> Chart/21(Fahrer_B)
  Estimate acceleration/9(Beschleunigung_400) -> Chart/10(Beschleunigung_400)
  Estimate acceleration/10(Beschleunigung_450) -> Chart/11(Beschleunigung_450)
  Fahrer_M/1 -> Chart/20(Fahrer_M)
  Demux/2 -> Estimate acceleration/3(Steigung_50)
  Demux/3 -> Chart/13(Steigung_100), Estimate acceleration/4(Steigung_100)
  Demux/4 -> Estimate acceleration/5(Steigung_150)
  Demux/5 -> Chart/14(Steigung_200), Estimate acceleration/6(Steigung_200)
  Demux/6 -> Estimate acceleration/7("Steigung_250 ")
  Demux/7 -> Chart/15(Steigung_300), Estimate acceleration/8(Steigung_300)
  Demux/8 -> Estimate acceleration/9(Steigung_350)
  Demux/9 -> Chart/16(Steigung_400), Estimate acceleration/10(Steigung_400)
  Demux/10 -> Estimate acceleration/11(Steigung_450)
  Demux/12 -> Chart/18(Steigung_distance_look_ahead_min)
  Demux/13 -> Chart/19(Steigung_distance_look_ahead_max)
  v_soll [m/s]/1 -> Braking from upper speed limit/1(v_soll [m/s]), Estimate critical slope/1(v_soll [m/s]), Estimate acceleration/1(v_soll [m/s])
  Memory2/1 -> Mux1/1
  Constant1/1 -> Switch1/1
  Constant2/1 -> Switch1/3
  Demux/11 -> Chart/17(Steigung_500)
  Switch1/1 -> Memory2/1
  Constant3/1 -> Sum1/1
  s_ist [m]/1 -> [s_ist], Sum3/2
  Sum1/1 -> altitude/1
  altitude/1 -> Demux1/1
  Demux1/2 -> Subtract/2
  Demux1/1 -> Subtract/1
  Subtract/1 -> Chart1/1(altitude_difference)
  Chart1/1(Signal_SOC_target_erhoehen) -> Memory3/1
  Integrator2/1 -> Chart1/2(Zurueckgelegte_Strecke)
  Constant/1 -> Switch/1
  Constant4/1 -> Switch/3
  Switch/1 -> addition_for_SOC/1
  Memory3/1 -> Switch/2, To Workspace/1, [Signal_SOC]
  Braking from upper speed limit/1(Out1) -> [Out1]
  [Out1] -> Mux1/3
  [Signal_Steigung] -> Integrator/2
  [Signal_SOC] -> Integrator2/2
  [Strecke] -> Chart/22(Zurueckgelegte_Strecke)
  Integrator/1 -> [Strecke]
  [Signal_Gefaelle] -> Integrator1/2
  Integrator1/1 -> [Strecke2]
  [Strecke2] -> Chart/23(Zurueckgelegte_Strecke_2)
  [s_ist] -> Sum1/2

== system HDVSim_XNG_Hybrid_Truck/Clutch ==
  note: Torque where clutch is disengaged
  note: Speed where clutch is engaged
  M_clutch_en [Nm] : Inport
  u_clutch : Inport  Port=2
  n_clutch_dis [rpm] : Inport  Port=3
  Compare To Zero : simulink/Logic and Bit Operations/Compare To Zero  relop===  OutDataTypeStr=boolean
  Constant : Constant  Value=0
  Idle engine speed : Constant  Value=engine.speed_min
  Logical Operator : Logic  AllPortsSameDT=off  OutDataTypeStr=boolean
  Lower engine speed : Saturate  UpperLimit=10000  LowerLimit=engine.speed_min  ZeroCross=off
  Switch1 : Switch  Threshold=1
  Switch2 : Switch  Threshold=1
  Switch3 : Switch  Threshold=1
  Zero1 : Constant  Value=0
  n_clutch_en [rpm] : Outport  VectorParamsAs1DForOutWhenUnconnected=off
  M_clutch_dis [Nm] : Outport  Port=2  VectorParamsAs1DForOutWhenUnconnected=off
  wires:
  n_clutch_dis [rpm]/1 -> Lower engine speed/1
  Lower engine speed/1 -> Switch3/3
  Switch2/1 -> Switch1/3
  Zero1/1 -> Switch2/1
  M_clutch_en [Nm]/1 -> Switch2/3
  [M_mot] -> Compare To Zero/1
  Compare To Zero/1 -> Logical Operator/1
  [ZKU] -> Logical Operator/2
  Logical Operator/1 -> Switch2/2
  Switch1/1 -> M_clutch_dis [Nm]/1
  Constant/1 -> Switch1/1
  Switch3/1 -> n_clutch_en [rpm]/1
  u_clutch/1 -> Switch3/2, Switch1/2
  Idle engine speed/1 -> Switch3/1

== system HDVSim_XNG_Hybrid_Truck/Differential ==
  note: Effective wheel torque
  M_Gearbox [Nm] : Inport
  n_tire [rpm] : Inport  Port=2
  Differential efficiency : Constant  Value=final_drive.trq_eff
  Differential gear ratio : Constant  Value=final_drive.ratio
  M' = M*i*eta : Product  Inputs=***
  Product : Product  Inputs=/  RndMeth=Zero
  Switch : Switch  ZeroCross=off
  n' *i = n : Product  RndMeth=Zero
  n_Gearbox [rpm] : Outport  VectorParamsAs1DForOutWhenUnconnected=off
  M_tire [Nm] : Outport  Port=2  VectorParamsAs1DForOutWhenUnconnected=off
  tag scope: Eta_Achsle_gearbox=global
  wires:
  M_Gearbox [Nm]/1 -> M' = M*i*eta/1, Switch/2
  n_tire [rpm]/1 -> n' *i = n/2
  Differential gear ratio/1 -> n' *i = n/1, M' = M*i*eta/3
  n' *i = n/1 -> n_Gearbox [rpm]/1
  M' = M*i*eta/1 -> M_tire [Nm]/1
  Differential efficiency/1 -> Product/1, Switch/1
  Product/1 -> Switch/3
  Switch/1 -> [Eta_Achsle_gearbox], M' = M*i*eta/2

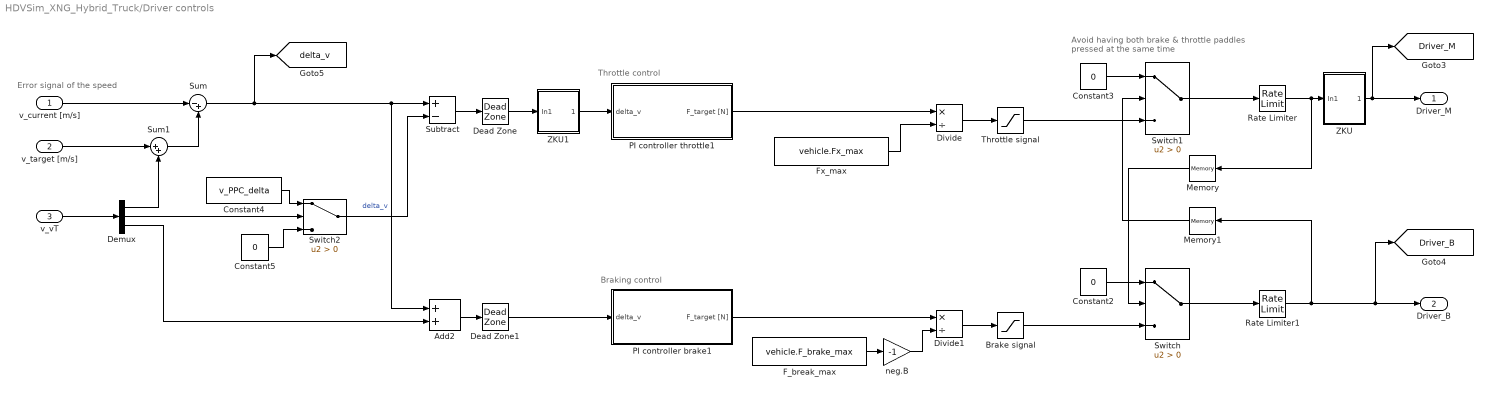
== system HDVSim_XNG_Hybrid_Truck/Driver controls ==
  note: Avoid having both brake & throttle
      | paddles pressed at the same time
  note: Throttle control
  note: Braking control
  note: Error signal of the speed
  v_current [m/s] : Inport
  v_target [m/s] : Inport  Port=2
  v_vT : Inport  Port=3
  Add2 : Sum
  Brake signal : Saturate  UpperLimit=1  LowerLimit=0  ZeroCross=off
  Constant2 : Constant  Value=0
  Constant3 : Constant  Value=0
  Constant4 : Constant  Value=v_PPC_delta
  Constant5 : Constant  Value=0
  Dead Zone : DeadZone  LowerValue=-0.1/3.6  UpperValue=0.1/3.6  ZeroCross=off  SaturateOnIntegerOverflow=on
  Dead Zone1 : DeadZone  LowerValue=-0.1/3.6  UpperValue=0  ZeroCross=off  SaturateOnIntegerOverflow=on
  Demux : Demux  Outputs=3
  Divide : Product  Inputs=*/
  Divide1 : Product  Inputs=*/
  F_break_max : Constant  Value=vehicle.F_brake_max
  Fx_max : Constant  Value=vehicle.Fx_max
  Memory : Memory
  Memory1 : Memory
  PI controller brake1 : Subsystem
  PI controller throttle1 : Subsystem
  Rate Limiter : RateLimiter  RisingSlewLimit=driver.slew_rate_throttle_in  FallingSlewLimit=driver.slew_rate_throttle_out  SampleTimeMode=inherited
  Rate Limiter1 : RateLimiter  RisingSlewLimit=driver.slew_rate_brake_in*100  FallingSlewLimit=driver.slew_rate_brake_out*100  SampleTimeMode=inherited
  Subtract : Sum  Inputs=+-
  Sum : Sum  Inputs=|-+
  Sum1 : Sum  Inputs=|++
  Switch : Switch  Criteria=u2 > Threshold
  Switch1 : Switch  Criteria=u2 > Threshold
  Switch2 : Switch  Criteria=u2 > Threshold
  Throttle signal : Saturate  UpperLimit=1  LowerLimit=0  ZeroCross=off
  ZKU : Subsystem
  ZKU1 : Subsystem
  neg.B : Gain  Gain=-1
  Driver_M : Outport  VectorParamsAs1DForOutWhenUnconnected=off
  Driver_B : Outport  Port=2  VectorParamsAs1DForOutWhenUnconnected=off
  tag scope: Driver_B=global, Driver_M=global, delta_v=global
  wires:
  Sum1/1 -> Sum/2
  v_current [m/s]/1 -> Sum/1
  Fx_max/1 -> Divide/2
  neg.B/1 -> Divide1/2
  Sum/1 -> [delta_v], Add2/1, Subtract/1  "delta_v"
  Divide1/1 -> Brake signal/1
  Divide/1 -> Throttle signal/1
  F_break_max/1 -> neg.B/1
  Switch/1 -> Rate Limiter1/1
  Brake signal/1 -> Switch/3
  Constant2/1 -> Switch/1
  Switch1/1 -> Rate Limiter/1
  Throttle signal/1 -> Switch1/3
  Rate Limiter1/1 -> Memory1/1, [Driver_B], Driver_B/1
  Rate Limiter/1 -> ZKU/1(In1), Memory/1
  Constant3/1 -> Switch1/1
  PI controller throttle1/1(F_target [N]) -> Divide/1
  Dead Zone/1 -> ZKU1/1(In1)
  Dead Zone1/1 -> PI controller brake1/1(delta_v)
  PI controller brake1/1(F_target [N]) -> Divide1/1
  ZKU/1(Out1) -> [Driver_M], Driver_M/1
  ZKU1/1(Out1) -> PI controller throttle1/1(delta_v)
  Memory/1 -> Switch/2
  Memory1/1 -> Switch1/2
  v_vT/1 -> Demux/1
  Switch2/1 -> Subtract/2
  Demux/2 -> Switch2/2
  Constant4/1 -> Switch2/1
  Constant5/1 -> Switch2/3
  Subtract/1 -> Dead Zone/1
  Demux/1 -> Sum1/2
  v_target [m/s]/1 -> Sum1/1
  Demux/3 -> Add2/2
  Add2/1 -> Dead Zone1/1

== system HDVSim_XNG_Hybrid_Truck/Driving cycle ==
  note: Lookup tables
  s_current [m] : Inport
  Clock : Clock
  Compare To Constant : simulink/Logic and Bit Operations/Compare To Constant  relop=>=  const=max_distance  OutDataTypeStr=boolean
  Constant : Constant  Value=0
  Holding time at the beginning : Subsystem
  Holding time at the end : Subsystem
  Significant speed deviation : Subsystem
  Slope-> angle : Trigonometry  Operator=atan
  Stop Simulation : Stop
  Stop Simulation 2 : Stop
  Switch : Switch  Criteria=u2 > Threshold
  Terminator : Terminator
  altitude : Lookup_n-D  NumberOfTableDimensions=1  BreakpointsForDimension1=cycle.distance  Table=cycle.altitude  InterpMethod=Flat  RndMeth=Simplest  InputSameDT=on
  slope : Lookup_n-D  NumberOfTableDimensions=1  BreakpointsForDimension1=cycle.distance  Table=cycle.slope  InterpMethod=Flat  RndMeth=Simplest  InputSameDT=on
  speed : Lookup_n-D  NumberOfTableDimensions=1  BreakpointsForDimension1=cycle.distance  Table=cycle.speed  InterpMethod=Flat  RndMeth=Simplest  InputSameDT=on
  stop_time : Lookup_n-D  NumberOfTableDimensions=1  BreakpointsForDimension1=cycle.distance  Table=cycle.stop_time  InterpMethod=Flat  RndMeth=Simplest  InputSameDT=on
  v_target [m/s] : Outport  VectorParamsAs1DForOutWhenUnconnected=off
  stop_time [s] : Outport  Port=2  VectorParamsAs1DForOutWhenUnconnected=off
  alpha [rad] : Outport  Port=3  VectorParamsAs1DForOutWhenUnconnected=off
  tag scope: altitude=global, slope_q=global, v_target_1=global
  wires:
  slope/1 -> Slope-> angle/1, [slope_q]
  Slope-> angle/1 -> alpha [rad]/1
  Compare To Constant/1 -> Holding time at the end/1(In1)
  Clock/1 -> Terminator/1  "duration"
  Switch/1 -> [v_target_1], v_target [m/s]/1
  s_current [m]/1 -> slope/1, Significant speed deviation/1(In1), Compare To Constant/1, altitude/1, speed/1, stop_time/1
  stop_time/1 -> stop_time [s]/1, Switch/2
  speed/1 -> Holding time at the beginning/1(In1)
  Holding time at the end/1(Out1) -> Stop Simulation/1
  Holding time at the beginning/1(Out1) -> Switch/3
  Constant/1 -> Switch/1
  altitude/1 -> [altitude]
  Significant speed deviation/1(Out1) -> Stop Simulation 2/1
